simulink model "r2_c8n856m1k_vacc_2b"  (R2012b)
settings: Solver=FixedStepDiscrete  SolverMode=SingleTasking  StartTime=0.0  StopTime=10000  RelTol=1e-3
undefined symbols (not defined in the file): Down, Up
libraries: xbsIndex_r4 x33706, casper_library_misc x90, casper_library_delays x13, casper_library_communications x8, xbsReplacements_r4 x3, xps_library x2
(part 63 of 265)

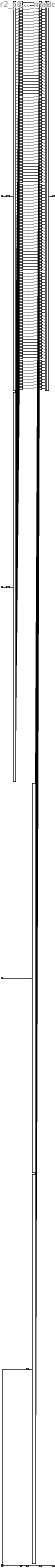
== system r2_c8n856m1k_vacc_2b/pfb/pfb_fir_generic/pfb_fir_taps/bus_madd ==
  a : Inport
  b : Inport  Port=2
  c : Inport  Port=3
  en : Inport  Port=4
  a*b+c_bussify : Subsystem  AncestorBlock=casper_library_flow_control/bus_create  mask(bus_create){inputNum=128}
      mask prompts: inputNum "Number of inputs:"
      mask init:
      | bus_create_init(gcb,...
      |     'inputNum', inputNum);
  a_debus : Subsystem  AncestorBlock=casper_library_flow_control/bus_expand  mask(bus_expand){mode=divisions of arbitrary size, outputNum=1, outputWidth=[10 10 10 10 10 10 10 10 10 10 10 10 10 10 10 10 10 10 10 10 10 10 10 10 10 10 10 10 10 10 10 10 10 10 10 10 10 10 10 10 10 10 10 10 10 10 10 10 10 10 10 10 10 10 10 10 10 10 10 10 10 10 10 10 10 10 10 10 10 10 10 10 10 10 10 10 10 10 10 10 10 10 10 10 10 10 10 10 10 10 10 10 10 10 10 10 10 10 10 10 10 10 10 10 10 10 10 10 10 10 10 10 10 10 10 10 10 10 10 10 10 10 10 10 10 10 10 10], outputBinaryPt=[9 9 9 9 9 9 9 9 9 9 9 9 9 9 9 9 9 9 9 9 9 9 9 9 9 9 9 9 9 9 9 9 9 9 9 9 9 9 9 9 9 9 9 9 9 9 9 9 9 9 9 9 9 9 9 9 9 9 9 9 9 9 9 9 9 9 9 9 9 9 9 9 9 9 9 9 9 9 9 9 9 9 9 9 9 9 9 9 9 9 9 9 9 9 9 9 9 9 9 9 9 9 9 9 9 9 9 9 9 9 9 9 9 9 9 9 9 9 9 9 9 9 9 9 9 9 9 9], outputArithmeticType=[1 1 1 1 1 1 1 1 1 1 1 1 1 1 1 1 1 1 1 1 1 1 1 1 1 1 1 1 1 1 1 1 1 1 1 1 1 1 1 1 1 1 1 1 1 1 1 1 1 1 1 1 1 1 1 1 1 1 1 1 1 1 1 1 1 1 1 1 1 1 1 1 1 1 1 1 1 1 1 1 1 1 1 1 1 1 1 1 1 1 1 1 1 1 1 1 1 1 1 1 1 1 1 1 1 1 1 1 1 1 1 1 1 1 1 1 1 1 1 1 1 1 1 1 1 1 1 1], show_format=on, outputToWorkspace=off, variablePrefix=, outputToModelAsWell=on}
      mask prompts: outputNum "Number of outputs:"; outputWidth "Output width [msb ... lsb]:"; outputBinaryPt "Output binary point position [msb ... lsb]:"; outputArithmeticType "Output arithmetic type (ufix=0, fix=1, bool=2) [msb ... lsb]:"; show_format "Print format string?"; outputToWorkspace "Output to workspace?"; variablePrefix "Variable name prefix:"; outputToModelAsWell "Output to model as well?"
      mask help:
      | Expands a 'bus' made using a bus_create or Xilinx
      | concat block.
      | The input will be divided into the specified number
      | of outputs with the specified arithmetic types.
      | Divisions are made from most significant bit.
      mask init: same as r2_c8n856m1k_vacc_2b/bus_expand15
      settings: AttributesFormatString=128 outputs: f10.9,f10.9,f10.9,f10.9,f10.9,f10.9,f10.9,f10.9,f10.9,f10.9,f10.9,f10.9,f10.9,f10.9,f10.9,f10.9,f10.9,f10.9,f10.9,f10.9,f10.9,f10.9,f10.9,f10.9,f10.9,f10.9,f10.9,f10.9,f10.9,f10.9,f10.9,f10.9,f10.9,f10.9,f10.9,f10.9,f10.9,f10.9,f10.9,f10.9,f10.9,f10.9,f10.9,f10.9,f10.9,f10.9,f10.9,f10.9,f10.9,f10.9,f10.9,f10.9,f10.9,f10.9,f10.9,f10.9,f10.9,f10.9,f10.9,f10.9,f10.9,f10.9,f10.9,f10.9,f10.9,f10.9,f10.9,f10.9,f10.9,f10.9,f10.9,f10.9,f10.9,f10.9,f10.9,f10.9,f10.9,f10.9,f10.9,f10.9,f10.9,f10.9,f10.9,f10.9,f10.9,f10.9,f10.9,f10.9,f10.9,f10.9,f10.9,f10.9,f10.9,f10.9,f10.9,f10.9,f10.9,f10.9,f10.9,f10.9,f10.9,f10.9,f10.9,f10.9,f10.9,f10.9,f10.9,f10.9,f10.9,f10.9,f10.9,f10.9,f10.9,f10.9,f10.9,f10.9,f10.9,f10.9,f10.9,f10.9,f10.9,f10.9,f10.9,f10.9,f10.9,f10.9,f10.9,f10.9,
      mask TypeOptions mode: divisions of equal size | divisions of arbitrary size
      mask Callback mode: bus_expand_callback();
      mask Callback outputWidth: bus_expand_callback();
      mask Callback outputToWorkspace: bus_expand_callback();
  addsub{1..16} (16 alike) : xbsIndex_r4/AddSub  mode=Addition  use_carryin=off  use_carryout=off  en=on  latency=2  precision=User Defined  arith_type="Signed  (2's comp)"  n_bits=28  bin_pt=26  quantization=Truncate  overflow=Wrap  dbl_ovrd=off  use_behavioral_HDL=on  hw_selection=Fabric  pipelined=on  block_type=addsub
  addsub{97..112} (16 alike) : xbsIndex_r4/AddSub  mode=Addition  use_carryin=off  use_carryout=off  en=on  latency=2  precision=User Defined  arith_type="Signed  (2's comp)"  n_bits=34  bin_pt=26  quantization=Truncate  overflow=Wrap  dbl_ovrd=off  use_behavioral_HDL=on  hw_selection=Fabric  pipelined=on  block_type=addsub
  addsub{113..128} (16 alike) : xbsIndex_r4/AddSub  mode=Addition  use_carryin=off  use_carryout=off  en=on  latency=2  precision=User Defined  arith_type="Signed  (2's comp)"  n_bits=35  bin_pt=26  quantization=Truncate  overflow=Wrap  dbl_ovrd=off  use_behavioral_HDL=on  hw_selection=Fabric  pipelined=on  block_type=addsub
  addsub{17..32} (16 alike) : xbsIndex_r4/AddSub  mode=Addition  use_carryin=off  use_carryout=off  en=on  latency=2  precision=User Defined  arith_type="Signed  (2's comp)"  n_bits=29  bin_pt=26  quantization=Truncate  overflow=Wrap  dbl_ovrd=off  use_behavioral_HDL=on  hw_selection=Fabric  pipelined=on  block_type=addsub
  addsub{33..48} (16 alike) : xbsIndex_r4/AddSub  mode=Addition  use_carryin=off  use_carryout=off  en=on  latency=2  precision=User Defined  arith_type="Signed  (2's comp)"  n_bits=30  bin_pt=26  quantization=Truncate  overflow=Wrap  dbl_ovrd=off  use_behavioral_HDL=on  hw_selection=Fabric  pipelined=on  block_type=addsub
  addsub{49..64} (16 alike) : xbsIndex_r4/AddSub  mode=Addition  use_carryin=off  use_carryout=off  en=on  latency=2  precision=User Defined  arith_type="Signed  (2's comp)"  n_bits=31  bin_pt=26  quantization=Truncate  overflow=Wrap  dbl_ovrd=off  use_behavioral_HDL=on  hw_selection=Fabric  pipelined=on  block_type=addsub
  addsub{65..80} (16 alike) : xbsIndex_r4/AddSub  mode=Addition  use_carryin=off  use_carryout=off  en=on  latency=2  precision=User Defined  arith_type="Signed  (2's comp)"  n_bits=32  bin_pt=26  quantization=Truncate  overflow=Wrap  dbl_ovrd=off  use_behavioral_HDL=on  hw_selection=Fabric  pipelined=on  block_type=addsub
  addsub{81..96} (16 alike) : xbsIndex_r4/AddSub  mode=Addition  use_carryin=off  use_carryout=off  en=on  latency=2  precision=User Defined  arith_type="Signed  (2's comp)"  n_bits=33  bin_pt=26  quantization=Truncate  overflow=Wrap  dbl_ovrd=off  use_behavioral_HDL=on  hw_selection=Fabric  pipelined=on  block_type=addsub
  b_debus : Subsystem  AncestorBlock=casper_library_flow_control/bus_expand  mask(bus_expand){mode=divisions of arbitrary size, outputNum=1, outputWidth=[18 18 18 18 18 18 18 18 18 18 18 18 18 18 18 18 18 18 18 18 18 18 18 18 18 18 18 18 18 18 18 18 18 18 18 18 18 18 18 18 18 18 18 18 18 18 18 18 18 18 18 18 18 18 18 18 18 18 18 18 18 18 18 18 18 18 18 18 18 18 18 18 18 18 18 18 18 18 18 18 18 18 18 18 18 18 18 18 18 18 18 18 18 18 18 18 18 18 18 18 18 18 18 18 18 18 18 18 18 18 18 18 18 18 18 18 18 18 18 18 18 18 18 18 18 18 18 18], outputBinaryPt=[17 17 17 17 17 17 17 17 17 17 17 17 17 17 17 17 17 17 17 17 17 17 17 17 17 17 17 17 17 17 17 17 17 17 17 17 17 17 17 17 17 17 17 17 17 17 17 17 17 17 17 17 17 17 17 17 17 17 17 17 17 17 17 17 17 17 17 17 17 17 17 17 17 17 17 17 17 17 17 17 17 17 17 17 17 17 17 17 17 17 17 17 17 17 17 17 17 17 17 17 17 17 17 17 17 17 17 17 17 17 17 17 17 17 17 17 17 17 17 17 17 17 17 17 17 17 17 17], outputArithmeticType=[1 1 1 1 1 1 1 1 1 1 1 1 1 1 1 1 1 1 1 1 1 1 1 1 1 1 1 1 1 1 1 1 1 1 1 1 1 1 1 1 1 1 1 1 1 1 1 1 1 1 1 1 1 1 1 1 1 1 1 1 1 1 1 1 1 1 1 1 1 1 1 1 1 1 1 1 1 1 1 1 1 1 1 1 1 1 1 1 1 1 1 1 1 1 1 1 1 1 1 1 1 1 1 1 1 1 1 1 1 1 1 1 1 1 1 1 1 1 1 1 1 1 1 1 1 1 1 1], show_format=on, outputToWorkspace=off, variablePrefix=, outputToModelAsWell=on}
      mask prompts: outputNum "Number of outputs:"; outputWidth "Output width [msb ... lsb]:"; outputBinaryPt "Output binary point position [msb ... lsb]:"; outputArithmeticType "Output arithmetic type (ufix=0, fix=1, bool=2) [msb ... lsb]:"; show_format "Print format string?"; outputToWorkspace "Output to workspace?"; variablePrefix "Variable name prefix:"; outputToModelAsWell "Output to model as well?"
      mask help:
      | Expands a 'bus' made using a bus_create or Xilinx
      | concat block.
      | The input will be divided into the specified number
      | of outputs with the specified arithmetic types.
      | Divisions are made from most significant bit.
      mask init: same as r2_c8n856m1k_vacc_2b/bus_expand15
      settings: AttributesFormatString=128 outputs: f18.17,f18.17,f18.17,f18.17,f18.17,f18.17,f18.17,f18.17,f18.17,f18.17,f18.17,f18.17,f18.17,f18.17,f18.17,f18.17,f18.17,f18.17,f18.17,f18.17,f18.17,f18.17,f18.17,f18.17,f18.17,f18.17,f18.17,f18.17,f18.17,f18.17,f18.17,f18.17,f18.17,f18.17,f18.17,f18.17,f18.17,f18.17,f18.17,f18.17,f18.17,f18.17,f18.17,f18.17,f18.17,f18.17,f18.17,f18.17,f18.17,f18.17,f18.17,f18.17,f18.17,f18.17,f18.17,f18.17,f18.17,f18.17,f18.17,f18.17,f18.17,f18.17,f18.17,f18.17,f18.17,f18.17,f18.17,f18.17,f18.17,f18.17,f18.17,f18.17,f18.17,f18.17,f18.17,f18.17,f18.17,f18.17,f18.17,f18.17,f18.17,f18.17,f18.17,f18.17,f18.17,f18.17,f18.17,f18.17,f18.17,f18.17,f18.17,f18.17,f18.17,f18.17,f18.17,f18.17,f18.17,f18.17,f18.17,f18.17,f18.17,f18.17,f18.17,f18.17,f18.17,f18.17,f18.17,f18.17,f18.17,f18.17,f18.17,f18.17,f18.17,f18.17,f18.17,f18.17,f18.17,f18.17,f18.17,f18.17,f18.17,f18.17,f18.17,f18.17,f18.17,f18.17,f18.17,f18.17,
      mask TypeOptions mode: divisions of equal size | divisions of arbitrary size
      mask Callback mode: bus_expand_callback();
      mask Callback outputWidth: bus_expand_callback();
      mask Callback outputToWorkspace: bus_expand_callback();
  c_debus : Subsystem  AncestorBlock=casper_library_flow_control/bus_expand  mask(bus_expand){mode=divisions of arbitrary size, outputNum=1, outputWidth=[28 28 28 28 28 28 28 28 28 28 28 28 28 28 28 28 28 28 28 28 28 28 28 28 28 28 28 28 28 28 28 28 29 29 29 29 29 29 29 29 29 29 29 29 29 29 29 29 30 30 30 30 30 30 30 30 30 30 30 30 30 30 30 30 31 31 31 31 31 31 31 31 31 31 31 31 31 31 31 31 32 32 32 32 32 32 32 32 32 32 32 32 32 32 32 32 33 33 33 33 33 33 33 33 33 33 33 33 33 33 33 33 34 34 34 34 34 34 34 34 34 34 34 34 34 34 34 34], outputBinaryPt=[26 26 26 26 26 26 26 26 26 26 26 26 26 26 26 26 26 26 26 26 26 26 26 26 26 26 26 26 26 26 26 26 26 26 26 26 26 26 26 26 26 26 26 26 26 26 26 26 26 26 26 26 26 26 26 26 26 26 26 26 26 26 26 26 26 26 26 26 26 26 26 26 26 26 26 26 26 26 26 26 26 26 26 26 26 26 26 26 26 26 26 26 26 26 26 26 26 26 26 26 26 26 26 26 26 26 26 26 26 26 26 26 26 26 26 26 26 26 26 26 26 26 26 26 26 26 26 26], outputArithmeticType=[1 1 1 1 1 1 1 1 1 1 1 1 1 1 1 1 1 1 1 1 1 1 1 1 1 1 1 1 1 1 1 1 1 1 1 1 1 1 1 1 1 1 1 1 1 1 1 1 1 1 1 1 1 1 1 1 1 1 1 1 1 1 1 1 1 1 1 1 1 1 1 1 1 1 1 1 1 1 1 1 1 1 1 1 1 1 1 1 1 1 1 1 1 1 1 1 1 1 1 1 1 1 1 1 1 1 1 1 1 1 1 1 1 1 1 1 1 1 1 1 1 1 1 1 1 1 1 1], show_format=on, outputToWorkspace=off, variablePrefix=, outputToModelAsWell=on}
      mask prompts: outputNum "Number of outputs:"; outputWidth "Output width [msb ... lsb]:"; outputBinaryPt "Output binary point position [msb ... lsb]:"; outputArithmeticType "Output arithmetic type (ufix=0, fix=1, bool=2) [msb ... lsb]:"; show_format "Print format string?"; outputToWorkspace "Output to workspace?"; variablePrefix "Variable name prefix:"; outputToModelAsWell "Output to model as well?"
      mask help:
      | Expands a 'bus' made using a bus_create or Xilinx
      | concat block.
      | The input will be divided into the specified number
      | of outputs with the specified arithmetic types.
      | Divisions are made from most significant bit.
      mask init: same as r2_c8n856m1k_vacc_2b/bus_expand15
      settings: AttributesFormatString=128 outputs: f28.26,f28.26,f28.26,f28.26,f28.26,f28.26,f28.26,f28.26,f28.26,f28.26,f28.26,f28.26,f28.26,f28.26,f28.26,f28.26,f28.26,f28.26,f28.26,f28.26,f28.26,f28.26,f28.26,f28.26,f28.26,f28.26,f28.26,f28.26,f28.26,f28.26,f28.26,f28.26,f29.26,f29.26,f29.26,f29.26,f29.26,f29.26,f29.26,f29.26,f29.26,f29.26,f29.26,f29.26,f29.26,f29.26,f29.26,f29.26,f30.26,f30.26,f30.26,f30.26,f30.26,f30.26,f30.26,f30.26,f30.26,f30.26,f30.26,f30.26,f30.26,f30.26,f30.26,f30.26,f31.26,f31.26,f31.26,f31.26,f31.26,f31.26,f31.26,f31.26,f31.26,f31.26,f31.26,f31.26,f31.26,f31.26,f31.26,f31.26,f32.26,f32.26,f32.26,f32.26,f32.26,f32.26,f32.26,f32.26,f32.26,f32.26,f32.26,f32.26,f32.26,f32.26,f32.26,f32.26,f33.26,f33.26,f33.26,f33.26,f33.26,f33.26,f33.26,f33.26,f33.26,f33.26,f33.26,f33.26,f33.26,f33.26,f33.26,f33.26,f34.26,f34.26,f34.26,f34.26,f34.26,f34.26,f34.26,f34.26,f34.26,f34.26,f34.26,f34.26,f34.26,f34.26,f34.26,f34.26,
      mask TypeOptions mode: divisions of equal size | divisions of arbitrary size
      mask Callback mode: bus_expand_callback();
      mask Callback outputWidth: bus_expand_callback();
      mask Callback outputToWorkspace: bus_expand_callback();
  den0 : xbsIndex_r4/Delay  rst=off  infoeditControl=Selection of Reset will increase slice count due to use of real FFs and instead of SRLs  en=off  latency=4  dbl_ovrd=off  reg_retiming=on  block_type=delay
  den1 : xbsIndex_r4/Delay  rst=off  infoeditControl=Selection of Reset will increase slice count due to use of real FFs and instead of SRLs  en=off  latency=1  dbl_ovrd=off  reg_retiming=on  block_type=delay
  den2 : xbsIndex_r4/Delay  rst=off  infoeditControl=Selection of Reset will increase slice count due to use of real FFs and instead of SRLs  en=off  latency=2  dbl_ovrd=off  reg_retiming=on  block_type=delay
  en_debus1 : Subsystem  AncestorBlock=casper_library_flow_control/bus_expand  mask(bus_expand){mode=divisions of equal size, outputNum=128, outputWidth=1, outputBinaryPt=0, outputArithmeticType=2, show_format=on, outputToWorkspace=off, variablePrefix=, outputToModelAsWell=on}
      mask prompts: outputNum "Number of outputs:"; outputWidth "Output width:"; outputBinaryPt "Output binary point position:"; outputArithmeticType "Output arithmetic type (ufix=0, fix=1, bool=2):"; show_format "Print format string?"; outputToWorkspace "Output to workspace?"; variablePrefix "Variable name prefix:"; outputToModelAsWell "Output to model as well?"
      mask help:
      | Expands a 'bus' made using a bus_create or Xilinx
      | concat block.
      | The input will be divided into the specified number
      | of outputs with the specified arithmetic types.
      | Divisions are made from most significant bit.
      mask init: same as r2_c8n856m1k_vacc_2b/bus_expand15
      settings: AttributesFormatString=128 outputs: b,b,b,b,b,b,b,b,b,b,b,b,b,b,b,b,b,b,b,b,b,b,b,b,b,b,b,b,b,b,b,b,b,b,b,b,b,b,b,b,b,b,b,b,b,b,b,b,b,b,b,b,b,b,b,b,b,b,b,b,b,b,b,b,b,b,b,b,b,b,b,b,b,b,b,b,b,b,b,b,b,b,b,b,b,b,b,b,b,b,b,b,b,b,b,b,b,b,b,b,b,b,b,b,b,b,b,b,b,b,b,b,b,b,b,b,b,b,b,b,b,b,b,b,b,b,b,b,
      mask TypeOptions mode: divisions of equal size | divisions of arbitrary size
      mask Callback mode: bus_expand_callback();
      mask Callback outputWidth: bus_expand_callback();
      mask Callback outputToWorkspace: bus_expand_callback();
  mult{1..128} (128 alike) : xbsIndex_r4/Mult  precision=Full  arith_type="Signed  (2's comp)"  n_bits=16  bin_pt=14  quantization=Truncate  overflow=Wrap  en=off  latency=4  dbl_ovrd=off  use_behavioral_HDL=on  opt=Speed  use_embedded=off  optimum_pipeline=off  pipeline=on  use_rpm=on  placement_style=Triangular  block_type=mult
  repa : Subsystem  AncestorBlock=casper_library_bus/bus_replicate  mask(bus_replicate){replication=1, latency=1, misc=off, implementation=core}
      mask prompts: replication "replication factor"; misc "misc support"; implementation "delay implementation"
      mask help: Output bus formed by replicating input bus a number of times
      mask init:
      | bus_replicate_init(gcb, ...
      |     'replication', replication, ...
      |     'latency', latency, ...
      |     'implementation', implementation, ...
      |     'misc', misc);
      mask TypeOptions implementation: core | behavioral
  repb : Subsystem  AncestorBlock=casper_library_bus/bus_replicate  mask(bus_replicate){replication=1, latency=1, misc=off, implementation=core}
      mask prompts: replication "replication factor"; misc "misc support"; implementation "delay implementation"
      mask help: Output bus formed by replicating input bus a number of times
      mask init:
      | bus_replicate_init(gcb, ...
      |     'replication', replication, ...
      |     'latency', latency, ...
      |     'implementation', implementation, ...
      |     'misc', misc);
      mask TypeOptions implementation: core | behavioral
  repen1 : Subsystem  AncestorBlock=casper_library_bus/bus_replicate  mask(bus_replicate){replication=128, latency=5, misc=off, implementation=core}
      mask prompts: replication "replication factor"; misc "misc support"; implementation "delay implementation"
      mask help: Output bus formed by replicating input bus a number of times
      mask init:
      | bus_replicate_init(gcb, ...
      |     'replication', replication, ...
      |     'latency', latency, ...
      |     'implementation', implementation, ...
      |     'misc', misc);
      mask TypeOptions implementation: core | behavioral
  a*b+c : Outport
  dvalid : Outport  Port=2
  wires:
  a/1 -> repa/1(in)
  b/1 -> repb/1(in)
  repa/1(out) -> a_debus/1(bus_in)
  repb/1(out) -> b_debus/1(bus_in)
  a_debus/1(msb_out128) -> mult1/1
  b_debus/1(msb_out128) -> mult1/2
  a_debus/2(out127) -> mult2/1
  b_debus/2(out127) -> mult2/2
  a_debus/3(out126) -> mult3/1
  b_debus/3(out126) -> mult3/2
  a_debus/4(out125) -> mult4/1
  b_debus/4(out125) -> mult4/2
  a_debus/5(out124) -> mult5/1
  b_debus/5(out124) -> mult5/2
  a_debus/6(out123) -> mult6/1
  b_debus/6(out123) -> mult6/2
  a_debus/7(out122) -> mult7/1
  b_debus/7(out122) -> mult7/2
  a_debus/8(out121) -> mult8/1
  b_debus/8(out121) -> mult8/2
  a_debus/9(out120) -> mult9/1
  b_debus/9(out120) -> mult9/2
  a_debus/10(out119) -> mult10/1
  b_debus/10(out119) -> mult10/2
  a_debus/11(out118) -> mult11/1
  b_debus/11(out118) -> mult11/2
  a_debus/12(out117) -> mult12/1
  b_debus/12(out117) -> mult12/2
  a_debus/13(out116) -> mult13/1
  b_debus/13(out116) -> mult13/2
  a_debus/14(out115) -> mult14/1
  b_debus/14(out115) -> mult14/2
  a_debus/15(out114) -> mult15/1
  b_debus/15(out114) -> mult15/2
  a_debus/16(out113) -> mult16/1
  b_debus/16(out113) -> mult16/2
  a_debus/17(out112) -> mult17/1
  b_debus/17(out112) -> mult17/2
  a_debus/18(out111) -> mult18/1
  b_debus/18(out111) -> mult18/2
  a_debus/19(out110) -> mult19/1
  b_debus/19(out110) -> mult19/2
  a_debus/20(out109) -> mult20/1
  b_debus/20(out109) -> mult20/2
  a_debus/21(out108) -> mult21/1
  b_debus/21(out108) -> mult21/2
  a_debus/22(out107) -> mult22/1
  b_debus/22(out107) -> mult22/2
  a_debus/23(out106) -> mult23/1
  b_debus/23(out106) -> mult23/2
  a_debus/24(out105) -> mult24/1
  b_debus/24(out105) -> mult24/2
  a_debus/25(out104) -> mult25/1
  b_debus/25(out104) -> mult25/2
  a_debus/26(out103) -> mult26/1
  b_debus/26(out103) -> mult26/2
  a_debus/27(out102) -> mult27/1
  b_debus/27(out102) -> mult27/2
  a_debus/28(out101) -> mult28/1
  b_debus/28(out101) -> mult28/2
  a_debus/29(out100) -> mult29/1
  b_debus/29(out100) -> mult29/2
  a_debus/30(out99) -> mult30/1
  b_debus/30(out99) -> mult30/2
  a_debus/31(out98) -> mult31/1
  b_debus/31(out98) -> mult31/2
  a_debus/32(out97) -> mult32/1
  b_debus/32(out97) -> mult32/2
  a_debus/33(out96) -> mult33/1
  b_debus/33(out96) -> mult33/2
  a_debus/34(out95) -> mult34/1
  b_debus/34(out95) -> mult34/2
  a_debus/35(out94) -> mult35/1
  b_debus/35(out94) -> mult35/2
  a_debus/36(out93) -> mult36/1
  b_debus/36(out93) -> mult36/2
  a_debus/37(out92) -> mult37/1
  b_debus/37(out92) -> mult37/2
  a_debus/38(out91) -> mult38/1
  b_debus/38(out91) -> mult38/2
  a_debus/39(out90) -> mult39/1
  b_debus/39(out90) -> mult39/2
  a_debus/40(out89) -> mult40/1
  b_debus/40(out89) -> mult40/2
  a_debus/41(out88) -> mult41/1
  b_debus/41(out88) -> mult41/2
  a_debus/42(out87) -> mult42/1
  b_debus/42(out87) -> mult42/2
  a_debus/43(out86) -> mult43/1
  b_debus/43(out86) -> mult43/2
  a_debus/44(out85) -> mult44/1
  b_debus/44(out85) -> mult44/2
  a_debus/45(out84) -> mult45/1
  b_debus/45(out84) -> mult45/2
  a_debus/46(out83) -> mult46/1
  b_debus/46(out83) -> mult46/2
  a_debus/47(out82) -> mult47/1
  b_debus/47(out82) -> mult47/2
  a_debus/48(out81) -> mult48/1
  b_debus/48(out81) -> mult48/2
  a_debus/49(out80) -> mult49/1
  b_debus/49(out80) -> mult49/2
  a_debus/50(out79) -> mult50/1
  b_debus/50(out79) -> mult50/2
  a_debus/51(out78) -> mult51/1
  b_debus/51(out78) -> mult51/2
  a_debus/52(out77) -> mult52/1
  b_debus/52(out77) -> mult52/2
  a_debus/53(out76) -> mult53/1
  b_debus/53(out76) -> mult53/2
  a_debus/54(out75) -> mult54/1
  b_debus/54(out75) -> mult54/2
  a_debus/55(out74) -> mult55/1
  b_debus/55(out74) -> mult55/2
  a_debus/56(out73) -> mult56/1
  b_debus/56(out73) -> mult56/2
  a_debus/57(out72) -> mult57/1
  b_debus/57(out72) -> mult57/2
  a_debus/58(out71) -> mult58/1
  b_debus/58(out71) -> mult58/2
  a_debus/59(out70) -> mult59/1
  b_debus/59(out70) -> mult59/2
  a_debus/60(out69) -> mult60/1
  b_debus/60(out69) -> mult60/2
  a_debus/61(out68) -> mult61/1
  b_debus/61(out68) -> mult61/2
  a_debus/62(out67) -> mult62/1
  b_debus/62(out67) -> mult62/2
  a_debus/63(out66) -> mult63/1
  b_debus/63(out66) -> mult63/2
  a_debus/64(out65) -> mult64/1
  b_debus/64(out65) -> mult64/2
  a_debus/65(out64) -> mult65/1
  b_debus/65(out64) -> mult65/2
  a_debus/66(out63) -> mult66/1
  b_debus/66(out63) -> mult66/2
  a_debus/67(out62) -> mult67/1
  b_debus/67(out62) -> mult67/2
  a_debus/68(out61) -> mult68/1
  b_debus/68(out61) -> mult68/2
  a_debus/69(out60) -> mult69/1
  b_debus/69(out60) -> mult69/2
  a_debus/70(out59) -> mult70/1
  b_debus/70(out59) -> mult70/2
  a_debus/71(out58) -> mult71/1
  b_debus/71(out58) -> mult71/2
  a_debus/72(out57) -> mult72/1
  b_debus/72(out57) -> mult72/2
  a_debus/73(out56) -> mult73/1
  b_debus/73(out56) -> mult73/2
  a_debus/74(out55) -> mult74/1
  b_debus/74(out55) -> mult74/2
  a_debus/75(out54) -> mult75/1
  b_debus/75(out54) -> mult75/2
  a_debus/76(out53) -> mult76/1
  b_debus/76(out53) -> mult76/2
  a_debus/77(out52) -> mult77/1
  b_debus/77(out52) -> mult77/2
  a_debus/78(out51) -> mult78/1
  b_debus/78(out51) -> mult78/2
  a_debus/79(out50) -> mult79/1
  b_debus/79(out50) -> mult79/2
  a_debus/80(out49) -> mult80/1
  b_debus/80(out49) -> mult80/2
  a_debus/81(out48) -> mult81/1
  b_debus/81(out48) -> mult81/2
  a_debus/82(out47) -> mult82/1
  b_debus/82(out47) -> mult82/2
  a_debus/83(out46) -> mult83/1
  b_debus/83(out46) -> mult83/2
  a_debus/84(out45) -> mult84/1
  b_debus/84(out45) -> mult84/2
  a_debus/85(out44) -> mult85/1
  b_debus/85(out44) -> mult85/2
  a_debus/86(out43) -> mult86/1
  b_debus/86(out43) -> mult86/2
  a_debus/87(out42) -> mult87/1
  b_debus/87(out42) -> mult87/2
  a_debus/88(out41) -> mult88/1
  b_debus/88(out41) -> mult88/2
  a_debus/89(out40) -> mult89/1
  b_debus/89(out40) -> mult89/2
  a_debus/90(out39) -> mult90/1
  b_debus/90(out39) -> mult90/2
  a_debus/91(out38) -> mult91/1
  b_debus/91(out38) -> mult91/2
  a_debus/92(out37) -> mult92/1
  b_debus/92(out37) -> mult92/2
  a_debus/93(out36) -> mult93/1
  b_debus/93(out36) -> mult93/2
  a_debus/94(out35) -> mult94/1
  b_debus/94(out35) -> mult94/2
  a_debus/95(out34) -> mult95/1
  b_debus/95(out34) -> mult95/2
  a_debus/96(out33) -> mult96/1
  b_debus/96(out33) -> mult96/2
  a_debus/97(out32) -> mult97/1
  b_debus/97(out32) -> mult97/2
  a_debus/98(out31) -> mult98/1
  b_debus/98(out31) -> mult98/2
  a_debus/99(out30) -> mult99/1
  b_debus/99(out30) -> mult99/2
  a_debus/100(out29) -> mult100/1
  b_debus/100(out29) -> mult100/2
  a_debus/101(out28) -> mult101/1
  b_debus/101(out28) -> mult101/2
  a_debus/102(out27) -> mult102/1
  b_debus/102(out27) -> mult102/2
  a_debus/103(out26) -> mult103/1
  b_debus/103(out26) -> mult103/2
  a_debus/104(out25) -> mult104/1
  b_debus/104(out25) -> mult104/2
  a_debus/105(out24) -> mult105/1
  b_debus/105(out24) -> mult105/2
  a_debus/106(out23) -> mult106/1
  b_debus/106(out23) -> mult106/2
  a_debus/107(out22) -> mult107/1
  b_debus/107(out22) -> mult107/2
  a_debus/108(out21) -> mult108/1
  b_debus/108(out21) -> mult108/2
  a_debus/109(out20) -> mult109/1
  b_debus/109(out20) -> mult109/2
  a_debus/110(out19) -> mult110/1
  b_debus/110(out19) -> mult110/2
  a_debus/111(out18) -> mult111/1
  b_debus/111(out18) -> mult111/2
  a_debus/112(out17) -> mult112/1
  b_debus/112(out17) -> mult112/2
  a_debus/113(out16) -> mult113/1
  b_debus/113(out16) -> mult113/2
  a_debus/114(out15) -> mult114/1
  b_debus/114(out15) -> mult114/2
  a_debus/115(out14) -> mult115/1
  b_debus/115(out14) -> mult115/2
  a_debus/116(out13) -> mult116/1
  b_debus/116(out13) -> mult116/2
  a_debus/117(out12) -> mult117/1
  b_debus/117(out12) -> mult117/2
  a_debus/118(out11) -> mult118/1
  b_debus/118(out11) -> mult118/2
  a_debus/119(out10) -> mult119/1
  b_debus/119(out10) -> mult119/2
  a_debus/120(out9) -> mult120/1
  b_debus/120(out9) -> mult120/2
  a_debus/121(out8) -> mult121/1
  b_debus/121(out8) -> mult121/2
  a_debus/122(out7) -> mult122/1
  b_debus/122(out7) -> mult122/2
  a_debus/123(out6) -> mult123/1
  b_debus/123(out6) -> mult123/2
  a_debus/124(out5) -> mult124/1
  b_debus/124(out5) -> mult124/2
  a_debus/125(out4) -> mult125/1
  b_debus/125(out4) -> mult125/2
  a_debus/126(out3) -> mult126/1
  b_debus/126(out3) -> mult126/2
  a_debus/127(out2) -> mult127/1
  b_debus/127(out2) -> mult127/2
  a_debus/128(lsb_out1) -> mult128/1
  b_debus/128(lsb_out1) -> mult128/2
  en/1 -> den0/1, repen1/1(in)
  den0/1 -> den1/1
  c/1 -> c_debus/1(bus_in)
  repen1/1(out) -> en_debus1/1(bus_in)
  mult1/1 -> addsub1/1
  c_debus/1(msb_out128) -> addsub1/2
  en_debus1/1(msb_out128) -> addsub1/3
  mult2/1 -> addsub2/1
  c_debus/2(out127) -> addsub2/2
  en_debus1/2(out127) -> addsub2/3
  mult3/1 -> addsub3/1
  c_debus/3(out126) -> addsub3/2
  en_debus1/3(out126) -> addsub3/3
  mult4/1 -> addsub4/1
  c_debus/4(out125) -> addsub4/2
  en_debus1/4(out125) -> addsub4/3
  mult5/1 -> addsub5/1
  c_debus/5(out124) -> addsub5/2
  en_debus1/5(out124) -> addsub5/3
  mult6/1 -> addsub6/1
  c_debus/6(out123) -> addsub6/2
  en_debus1/6(out123) -> addsub6/3
  mult7/1 -> addsub7/1
  c_debus/7(out122) -> addsub7/2
  en_debus1/7(out122) -> addsub7/3
  mult8/1 -> addsub8/1
  c_debus/8(out121) -> addsub8/2
  en_debus1/8(out121) -> addsub8/3
  mult9/1 -> addsub9/1
  c_debus/9(out120) -> addsub9/2
  en_debus1/9(out120) -> addsub9/3
  mult10/1 -> addsub10/1
  c_debus/10(out119) -> addsub10/2
  en_debus1/10(out119) -> addsub10/3
  mult11/1 -> addsub11/1
  c_debus/11(out118) -> addsub11/2
  en_debus1/11(out118) -> addsub11/3
  mult12/1 -> addsub12/1
  c_debus/12(out117) -> addsub12/2
  en_debus1/12(out117) -> addsub12/3
  mult13/1 -> addsub13/1
  c_debus/13(out116) -> addsub13/2
  en_debus1/13(out116) -> addsub13/3
  mult14/1 -> addsub14/1
  c_debus/14(out115) -> addsub14/2
  en_debus1/14(out115) -> addsub14/3
  mult15/1 -> addsub15/1
  c_debus/15(out114) -> addsub15/2
  en_debus1/15(out114) -> addsub15/3
  mult16/1 -> addsub16/1
  c_debus/16(out113) -> addsub16/2
  en_debus1/16(out113) -> addsub16/3
  mult17/1 -> addsub17/1
  c_debus/17(out112) -> addsub17/2
  en_debus1/17(out112) -> addsub17/3
  mult18/1 -> addsub18/1
  c_debus/18(out111) -> addsub18/2
  en_debus1/18(out111) -> addsub18/3
  mult19/1 -> addsub19/1
  c_debus/19(out110) -> addsub19/2
  en_debus1/19(out110) -> addsub19/3
  mult20/1 -> addsub20/1
  c_debus/20(out109) -> addsub20/2
  en_debus1/20(out109) -> addsub20/3
  mult21/1 -> addsub21/1
  c_debus/21(out108) -> addsub21/2
  en_debus1/21(out108) -> addsub21/3
  mult22/1 -> addsub22/1
  c_debus/22(out107) -> addsub22/2
  en_debus1/22(out107) -> addsub22/3
  mult23/1 -> addsub23/1
  c_debus/23(out106) -> addsub23/2
  en_debus1/23(out106) -> addsub23/3
  mult24/1 -> addsub24/1
  c_debus/24(out105) -> addsub24/2
  en_debus1/24(out105) -> addsub24/3
  mult25/1 -> addsub25/1
  c_debus/25(out104) -> addsub25/2
  en_debus1/25(out104) -> addsub25/3
  mult26/1 -> addsub26/1
  c_debus/26(out103) -> addsub26/2
  en_debus1/26(out103) -> addsub26/3
  mult27/1 -> addsub27/1
  c_debus/27(out102) -> addsub27/2
  en_debus1/27(out102) -> addsub27/3
  mult28/1 -> addsub28/1
  c_debus/28(out101) -> addsub28/2
  en_debus1/28(out101) -> addsub28/3
  mult29/1 -> addsub29/1
  c_debus/29(out100) -> addsub29/2
  en_debus1/29(out100) -> addsub29/3
  mult30/1 -> addsub30/1
  c_debus/30(out99) -> addsub30/2
  en_debus1/30(out99) -> addsub30/3
  mult31/1 -> addsub31/1
  c_debus/31(out98) -> addsub31/2
  en_debus1/31(out98) -> addsub31/3
  mult32/1 -> addsub32/1
  c_debus/32(out97) -> addsub32/2
  en_debus1/32(out97) -> addsub32/3
  mult33/1 -> addsub33/1
  c_debus/33(out96) -> addsub33/2
  en_debus1/33(out96) -> addsub33/3
  mult34/1 -> addsub34/1
  c_debus/34(out95) -> addsub34/2
  en_debus1/34(out95) -> addsub34/3
  mult35/1 -> addsub35/1
  c_debus/35(out94) -> addsub35/2
  en_debus1/35(out94) -> addsub35/3
  mult36/1 -> addsub36/1
  c_debus/36(out93) -> addsub36/2
  en_debus1/36(out93) -> addsub36/3
  mult37/1 -> addsub37/1
  c_debus/37(out92) -> addsub37/2
  en_debus1/37(out92) -> addsub37/3
  mult38/1 -> addsub38/1
  c_debus/38(out91) -> addsub38/2
  en_debus1/38(out91) -> addsub38/3
  mult39/1 -> addsub39/1
  c_debus/39(out90) -> addsub39/2
  en_debus1/39(out90) -> addsub39/3
  mult40/1 -> addsub40/1
  c_debus/40(out89) -> addsub40/2
  en_debus1/40(out89) -> addsub40/3
  mult41/1 -> addsub41/1
  c_debus/41(out88) -> addsub41/2
  en_debus1/41(out88) -> addsub41/3
  mult42/1 -> addsub42/1
  c_debus/42(out87) -> addsub42/2
  en_debus1/42(out87) -> addsub42/3
  mult43/1 -> addsub43/1
  c_debus/43(out86) -> addsub43/2
  en_debus1/43(out86) -> addsub43/3
  mult44/1 -> addsub44/1
  c_debus/44(out85) -> addsub44/2
  en_debus1/44(out85) -> addsub44/3
  mult45/1 -> addsub45/1
  c_debus/45(out84) -> addsub45/2
  en_debus1/45(out84) -> addsub45/3
  mult46/1 -> addsub46/1
  c_debus/46(out83) -> addsub46/2
  en_debus1/46(out83) -> addsub46/3
  mult47/1 -> addsub47/1
  c_debus/47(out82) -> addsub47/2
  en_debus1/47(out82) -> addsub47/3
  mult48/1 -> addsub48/1
  c_debus/48(out81) -> addsub48/2
  en_debus1/48(out81) -> addsub48/3
  mult49/1 -> addsub49/1
  c_debus/49(out80) -> addsub49/2
  en_debus1/49(out80) -> addsub49/3
  mult50/1 -> addsub50/1
  c_debus/50(out79) -> addsub50/2
  en_debus1/50(out79) -> addsub50/3
  mult51/1 -> addsub51/1
  c_debus/51(out78) -> addsub51/2
  en_debus1/51(out78) -> addsub51/3
  mult52/1 -> addsub52/1
  c_debus/52(out77) -> addsub52/2
  en_debus1/52(out77) -> addsub52/3
  mult53/1 -> addsub53/1
  c_debus/53(out76) -> addsub53/2
  en_debus1/53(out76) -> addsub53/3
  mult54/1 -> addsub54/1
  c_debus/54(out75) -> addsub54/2
  en_debus1/54(out75) -> addsub54/3
  mult55/1 -> addsub55/1
  c_debus/55(out74) -> addsub55/2
  en_debus1/55(out74) -> addsub55/3
  mult56/1 -> addsub56/1
  c_debus/56(out73) -> addsub56/2
  en_debus1/56(out73) -> addsub56/3
  mult57/1 -> addsub57/1
  c_debus/57(out72) -> addsub57/2
  en_debus1/57(out72) -> addsub57/3
  mult58/1 -> addsub58/1
  c_debus/58(out71) -> addsub58/2
  en_debus1/58(out71) -> addsub58/3
  mult59/1 -> addsub59/1
  c_debus/59(out70) -> addsub59/2
  en_debus1/59(out70) -> addsub59/3
  mult60/1 -> addsub60/1
  c_debus/60(out69) -> addsub60/2
  en_debus1/60(out69) -> addsub60/3
  mult61/1 -> addsub61/1
  c_debus/61(out68) -> addsub61/2
  en_debus1/61(out68) -> addsub61/3
  mult62/1 -> addsub62/1
  c_debus/62(out67) -> addsub62/2
  en_debus1/62(out67) -> addsub62/3
  mult63/1 -> addsub63/1
  c_debus/63(out66) -> addsub63/2
  en_debus1/63(out66) -> addsub63/3
  mult64/1 -> addsub64/1
  c_debus/64(out65) -> addsub64/2
  en_debus1/64(out65) -> addsub64/3
  mult65/1 -> addsub65/1
  c_debus/65(out64) -> addsub65/2
  en_debus1/65(out64) -> addsub65/3
  mult66/1 -> addsub66/1
  c_debus/66(out63) -> addsub66/2
  en_debus1/66(out63) -> addsub66/3
  mult67/1 -> addsub67/1
  c_debus/67(out62) -> addsub67/2
  en_debus1/67(out62) -> addsub67/3
  mult68/1 -> addsub68/1
  c_debus/68(out61) -> addsub68/2
  en_debus1/68(out61) -> addsub68/3
  mult69/1 -> addsub69/1
  c_debus/69(out60) -> addsub69/2
  en_debus1/69(out60) -> addsub69/3
  mult70/1 -> addsub70/1
  c_debus/70(out59) -> addsub70/2
  en_debus1/70(out59) -> addsub70/3
  mult71/1 -> addsub71/1
  c_debus/71(out58) -> addsub71/2
  en_debus1/71(out58) -> addsub71/3
  mult72/1 -> addsub72/1
  c_debus/72(out57) -> addsub72/2
  en_debus1/72(out57) -> addsub72/3
  mult73/1 -> addsub73/1
  c_debus/73(out56) -> addsub73/2
  en_debus1/73(out56) -> addsub73/3
  mult74/1 -> addsub74/1
  c_debus/74(out55) -> addsub74/2
  en_debus1/74(out55) -> addsub74/3
  mult75/1 -> addsub75/1
  c_debus/75(out54) -> addsub75/2
  en_debus1/75(out54) -> addsub75/3
  mult76/1 -> addsub76/1
  c_debus/76(out53) -> addsub76/2
  en_debus1/76(out53) -> addsub76/3
  mult77/1 -> addsub77/1
  c_debus/77(out52) -> addsub77/2
  en_debus1/77(out52) -> addsub77/3
  mult78/1 -> addsub78/1
  c_debus/78(out51) -> addsub78/2
  en_debus1/78(out51) -> addsub78/3
  mult79/1 -> addsub79/1
  c_debus/79(out50) -> addsub79/2
  en_debus1/79(out50) -> addsub79/3
  mult80/1 -> addsub80/1
  c_debus/80(out49) -> addsub80/2
  en_debus1/80(out49) -> addsub80/3
  mult81/1 -> addsub81/1
  c_debus/81(out48) -> addsub81/2
  en_debus1/81(out48) -> addsub81/3
  mult82/1 -> addsub82/1
  c_debus/82(out47) -> addsub82/2
  en_debus1/82(out47) -> addsub82/3
  mult83/1 -> addsub83/1
  c_debus/83(out46) -> addsub83/2
  en_debus1/83(out46) -> addsub83/3
  mult84/1 -> addsub84/1
  c_debus/84(out45) -> addsub84/2
  en_debus1/84(out45) -> addsub84/3
  mult85/1 -> addsub85/1
  c_debus/85(out44) -> addsub85/2
  en_debus1/85(out44) -> addsub85/3
  mult86/1 -> addsub86/1
  c_debus/86(out43) -> addsub86/2
  en_debus1/86(out43) -> addsub86/3
  mult87/1 -> addsub87/1
  c_debus/87(out42) -> addsub87/2
  en_debus1/87(out42) -> addsub87/3
  mult88/1 -> addsub88/1
  c_debus/88(out41) -> addsub88/2
  en_debus1/88(out41) -> addsub88/3
  mult89/1 -> addsub89/1
  c_debus/89(out40) -> addsub89/2
  en_debus1/89(out40) -> addsub89/3
  mult90/1 -> addsub90/1
  c_debus/90(out39) -> addsub90/2
  en_debus1/90(out39) -> addsub90/3
  mult91/1 -> addsub91/1
  c_debus/91(out38) -> addsub91/2
  en_debus1/91(out38) -> addsub91/3
  mult92/1 -> addsub92/1
  c_debus/92(out37) -> addsub92/2
  en_debus1/92(out37) -> addsub92/3
  mult93/1 -> addsub93/1
  c_debus/93(out36) -> addsub93/2
  en_debus1/93(out36) -> addsub93/3
  mult94/1 -> addsub94/1
  c_debus/94(out35) -> addsub94/2
  en_debus1/94(out35) -> addsub94/3
  mult95/1 -> addsub95/1
  c_debus/95(out34) -> addsub95/2
  en_debus1/95(out34) -> addsub95/3
  mult96/1 -> addsub96/1
  c_debus/96(out33) -> addsub96/2
  en_debus1/96(out33) -> addsub96/3
  mult97/1 -> addsub97/1
  c_debus/97(out32) -> addsub97/2
  en_debus1/97(out32) -> addsub97/3
  mult98/1 -> addsub98/1
  c_debus/98(out31) -> addsub98/2
  en_debus1/98(out31) -> addsub98/3
  mult99/1 -> addsub99/1
  c_debus/99(out30) -> addsub99/2
  en_debus1/99(out30) -> addsub99/3
  mult100/1 -> addsub100/1
  c_debus/100(out29) -> addsub100/2
  en_debus1/100(out29) -> addsub100/3
  mult101/1 -> addsub101/1
  c_debus/101(out28) -> addsub101/2
  en_debus1/101(out28) -> addsub101/3
  mult102/1 -> addsub102/1
  c_debus/102(out27) -> addsub102/2
  en_debus1/102(out27) -> addsub102/3
  mult103/1 -> addsub103/1
  c_debus/103(out26) -> addsub103/2
  en_debus1/103(out26) -> addsub103/3
  mult104/1 -> addsub104/1
  c_debus/104(out25) -> addsub104/2
  en_debus1/104(out25) -> addsub104/3
  mult105/1 -> addsub105/1
  c_debus/105(out24) -> addsub105/2
  en_debus1/105(out24) -> addsub105/3
  mult106/1 -> addsub106/1
  c_debus/106(out23) -> addsub106/2
  en_debus1/106(out23) -> addsub106/3
  mult107/1 -> addsub107/1
  c_debus/107(out22) -> addsub107/2
  en_debus1/107(out22) -> addsub107/3
  mult108/1 -> addsub108/1
  c_debus/108(out21) -> addsub108/2
  en_debus1/108(out21) -> addsub108/3
  mult109/1 -> addsub109/1
  c_debus/109(out20) -> addsub109/2
  en_debus1/109(out20) -> addsub109/3
  mult110/1 -> addsub110/1
  c_debus/110(out19) -> addsub110/2
  en_debus1/110(out19) -> addsub110/3
  mult111/1 -> addsub111/1
  c_debus/111(out18) -> addsub111/2
  en_debus1/111(out18) -> addsub111/3
  mult112/1 -> addsub112/1
  c_debus/112(out17) -> addsub112/2
  en_debus1/112(out17) -> addsub112/3
  mult113/1 -> addsub113/1
  c_debus/113(out16) -> addsub113/2
  en_debus1/113(out16) -> addsub113/3
  mult114/1 -> addsub114/1
  c_debus/114(out15) -> addsub114/2
  en_debus1/114(out15) -> addsub114/3
  mult115/1 -> addsub115/1
  c_debus/115(out14) -> addsub115/2
  en_debus1/115(out14) -> addsub115/3
  mult116/1 -> addsub116/1
  c_debus/116(out13) -> addsub116/2
  en_debus1/116(out13) -> addsub116/3
  mult117/1 -> addsub117/1
  c_debus/117(out12) -> addsub117/2
  en_debus1/117(out12) -> addsub117/3
  mult118/1 -> addsub118/1
  c_debus/118(out11) -> addsub118/2
  en_debus1/118(out11) -> addsub118/3
  mult119/1 -> addsub119/1
  c_debus/119(out10) -> addsub119/2
  en_debus1/119(out10) -> addsub119/3
  mult120/1 -> addsub120/1
  c_debus/120(out9) -> addsub120/2
  en_debus1/120(out9) -> addsub120/3
  mult121/1 -> addsub121/1
  c_debus/121(out8) -> addsub121/2
  en_debus1/121(out8) -> addsub121/3
  mult122/1 -> addsub122/1
  c_debus/122(out7) -> addsub122/2
  en_debus1/122(out7) -> addsub122/3
  mult123/1 -> addsub123/1
  c_debus/123(out6) -> addsub123/2
  en_debus1/123(out6) -> addsub123/3
  mult124/1 -> addsub124/1
  c_debus/124(out5) -> addsub124/2
  en_debus1/124(out5) -> addsub124/3
  mult125/1 -> addsub125/1
  c_debus/125(out4) -> addsub125/2
  en_debus1/125(out4) -> addsub125/3
  mult126/1 -> addsub126/1
  c_debus/126(out3) -> addsub126/2
  en_debus1/126(out3) -> addsub126/3
  mult127/1 -> addsub127/1
  c_debus/127(out2) -> addsub127/2
  en_debus1/127(out2) -> addsub127/3
  mult128/1 -> addsub128/1
  c_debus/128(lsb_out1) -> addsub128/2
  en_debus1/128(lsb_out1) -> addsub128/3
  den1/1 -> den2/1
  addsub1/1 -> a*b+c_bussify/1(in1)
  addsub2/1 -> a*b+c_bussify/2(in2)
  addsub3/1 -> a*b+c_bussify/3(in3)
  addsub4/1 -> a*b+c_bussify/4(in4)
  addsub5/1 -> a*b+c_bussify/5(in5)
  addsub6/1 -> a*b+c_bussify/6(in6)
  addsub7/1 -> a*b+c_bussify/7(in7)
  addsub8/1 -> a*b+c_bussify/8(in8)
  addsub9/1 -> a*b+c_bussify/9(in9)
  addsub10/1 -> a*b+c_bussify/10(in10)
  addsub11/1 -> a*b+c_bussify/11(in11)
  addsub12/1 -> a*b+c_bussify/12(in12)
  addsub13/1 -> a*b+c_bussify/13(in13)
  addsub14/1 -> a*b+c_bussify/14(in14)
  addsub15/1 -> a*b+c_bussify/15(in15)
  addsub16/1 -> a*b+c_bussify/16(in16)
  addsub17/1 -> a*b+c_bussify/17(in17)
  addsub18/1 -> a*b+c_bussify/18(in18)
  addsub19/1 -> a*b+c_bussify/19(in19)
  addsub20/1 -> a*b+c_bussify/20(in20)
  addsub21/1 -> a*b+c_bussify/21(in21)
  addsub22/1 -> a*b+c_bussify/22(in22)
  addsub23/1 -> a*b+c_bussify/23(in23)
  addsub24/1 -> a*b+c_bussify/24(in24)
  addsub25/1 -> a*b+c_bussify/25(in25)
  addsub26/1 -> a*b+c_bussify/26(in26)
  addsub27/1 -> a*b+c_bussify/27(in27)
  addsub28/1 -> a*b+c_bussify/28(in28)
  addsub29/1 -> a*b+c_bussify/29(in29)
  addsub30/1 -> a*b+c_bussify/30(in30)
  addsub31/1 -> a*b+c_bussify/31(in31)
  addsub32/1 -> a*b+c_bussify/32(in32)
  addsub33/1 -> a*b+c_bussify/33(in33)
  addsub34/1 -> a*b+c_bussify/34(in34)
  addsub35/1 -> a*b+c_bussify/35(in35)
  addsub36/1 -> a*b+c_bussify/36(in36)
  addsub37/1 -> a*b+c_bussify/37(in37)
  addsub38/1 -> a*b+c_bussify/38(in38)
  addsub39/1 -> a*b+c_bussify/39(in39)
  addsub40/1 -> a*b+c_bussify/40(in40)
  addsub41/1 -> a*b+c_bussify/41(in41)
  addsub42/1 -> a*b+c_bussify/42(in42)
  addsub43/1 -> a*b+c_bussify/43(in43)
  addsub44/1 -> a*b+c_bussify/44(in44)
  addsub45/1 -> a*b+c_bussify/45(in45)
  addsub46/1 -> a*b+c_bussify/46(in46)
  addsub47/1 -> a*b+c_bussify/47(in47)
  addsub48/1 -> a*b+c_bussify/48(in48)
  addsub49/1 -> a*b+c_bussify/49(in49)
  addsub50/1 -> a*b+c_bussify/50(in50)
  addsub51/1 -> a*b+c_bussify/51(in51)
  addsub52/1 -> a*b+c_bussify/52(in52)
  addsub53/1 -> a*b+c_bussify/53(in53)
  addsub54/1 -> a*b+c_bussify/54(in54)
  addsub55/1 -> a*b+c_bussify/55(in55)
  addsub56/1 -> a*b+c_bussify/56(in56)
  addsub57/1 -> a*b+c_bussify/57(in57)
  addsub58/1 -> a*b+c_bussify/58(in58)
  addsub59/1 -> a*b+c_bussify/59(in59)
  addsub60/1 -> a*b+c_bussify/60(in60)
  addsub61/1 -> a*b+c_bussify/61(in61)
  addsub62/1 -> a*b+c_bussify/62(in62)
  addsub63/1 -> a*b+c_bussify/63(in63)
  addsub64/1 -> a*b+c_bussify/64(in64)
  addsub65/1 -> a*b+c_bussify/65(in65)
  addsub66/1 -> a*b+c_bussify/66(in66)
  addsub67/1 -> a*b+c_bussify/67(in67)
  addsub68/1 -> a*b+c_bussify/68(in68)
  addsub69/1 -> a*b+c_bussify/69(in69)
  addsub70/1 -> a*b+c_bussify/70(in70)
  addsub71/1 -> a*b+c_bussify/71(in71)
  addsub72/1 -> a*b+c_bussify/72(in72)
  addsub73/1 -> a*b+c_bussify/73(in73)
  addsub74/1 -> a*b+c_bussify/74(in74)
  addsub75/1 -> a*b+c_bussify/75(in75)
  addsub76/1 -> a*b+c_bussify/76(in76)
  addsub77/1 -> a*b+c_bussify/77(in77)
  addsub78/1 -> a*b+c_bussify/78(in78)
  addsub79/1 -> a*b+c_bussify/79(in79)
  addsub80/1 -> a*b+c_bussify/80(in80)
  addsub81/1 -> a*b+c_bussify/81(in81)
  addsub82/1 -> a*b+c_bussify/82(in82)
  addsub83/1 -> a*b+c_bussify/83(in83)
  addsub84/1 -> a*b+c_bussify/84(in84)
  addsub85/1 -> a*b+c_bussify/85(in85)
  addsub86/1 -> a*b+c_bussify/86(in86)
  addsub87/1 -> a*b+c_bussify/87(in87)
  addsub88/1 -> a*b+c_bussify/88(in88)
  addsub89/1 -> a*b+c_bussify/89(in89)
  addsub90/1 -> a*b+c_bussify/90(in90)
  addsub91/1 -> a*b+c_bussify/91(in91)
  addsub92/1 -> a*b+c_bussify/92(in92)
  addsub93/1 -> a*b+c_bussify/93(in93)
  addsub94/1 -> a*b+c_bussify/94(in94)
  addsub95/1 -> a*b+c_bussify/95(in95)
  addsub96/1 -> a*b+c_bussify/96(in96)
  addsub97/1 -> a*b+c_bussify/97(in97)
  addsub98/1 -> a*b+c_bussify/98(in98)
  addsub99/1 -> a*b+c_bussify/99(in99)
  addsub100/1 -> a*b+c_bussify/100(in100)
  addsub101/1 -> a*b+c_bussify/101(in101)
  addsub102/1 -> a*b+c_bussify/102(in102)
  addsub103/1 -> a*b+c_bussify/103(in103)
  addsub104/1 -> a*b+c_bussify/104(in104)
  addsub105/1 -> a*b+c_bussify/105(in105)
  addsub106/1 -> a*b+c_bussify/106(in106)
  addsub107/1 -> a*b+c_bussify/107(in107)
  addsub108/1 -> a*b+c_bussify/108(in108)
  addsub109/1 -> a*b+c_bussify/109(in109)
  addsub110/1 -> a*b+c_bussify/110(in110)
  addsub111/1 -> a*b+c_bussify/111(in111)
  addsub112/1 -> a*b+c_bussify/112(in112)
  addsub113/1 -> a*b+c_bussify/113(in113)
  addsub114/1 -> a*b+c_bussify/114(in114)
  addsub115/1 -> a*b+c_bussify/115(in115)
  addsub116/1 -> a*b+c_bussify/116(in116)
  addsub117/1 -> a*b+c_bussify/117(in117)
  addsub118/1 -> a*b+c_bussify/118(in118)
  addsub119/1 -> a*b+c_bussify/119(in119)
  addsub120/1 -> a*b+c_bussify/120(in120)
  addsub121/1 -> a*b+c_bussify/121(in121)
  addsub122/1 -> a*b+c_bussify/122(in122)
  addsub123/1 -> a*b+c_bussify/123(in123)
  addsub124/1 -> a*b+c_bussify/124(in124)
  addsub125/1 -> a*b+c_bussify/125(in125)
  addsub126/1 -> a*b+c_bussify/126(in126)
  addsub127/1 -> a*b+c_bussify/127(in127)
  addsub128/1 -> a*b+c_bussify/128(in128)
  a*b+c_bussify/1(bus_out) -> a*b+c/1
  den2/1 -> dvalid/1
